simulink model "MX_Gateway"  (Simulink 9.0)
settings: Solver=FixedStepDiscrete  StartTime=0.0  StopTime=100  FixedStep=0.01  RelTol=1e-3
values from neighbouring files (symbols this model uses):
  ACTIVE = Bobcat.Parameter; CreateDefine( ACTIVE                         ,1                          ,'Define' ,'defines.h' )    [defines.m]
  ACTIVE_MODE_ONE = Bobcat.Parameter; CreateDefine( ACTIVE_MODE_ONE                ,19                         ,'Define' ,'defines.h' )    [defines.m]
  AUTHENTICATED = Bobcat.Parameter; CreateDefine( AUTHENTICATED                  ,1                          ,'Define' ,'defines.h' )    [defines.m]
  AUX_EMEA_CONTROLS = Bobcat.Parameter; CreateDefine( AUX_EMEA_CONTROLS  ,1   ,'Define' ,'defines.h' )    [enums.m]
  AUX_FLOW_HIGH = Bobcat.Parameter; CreateDefine( AUX_FLOW_HIGH           ,4  ,'Define' ,'defines.h' )    [enums.m]
  AUX_FLOW_INACTIVE = Bobcat.Parameter; CreateDefine( AUX_FLOW_INACTIVE       ,0  ,'Define' ,'defines.h' )    [enums.m]
  AUX_FLOW_LOW = Bobcat.Parameter; CreateDefine( AUX_FLOW_LOW            ,1  ,'Define' ,'defines.h' )    [enums.m]
  AUX_FLOW_MEDIUM = Bobcat.Parameter; CreateDefine( AUX_FLOW_MEDIUM         ,2  ,'Define' ,'defines.h' )    [enums.m]
  AUX_FLOW_STANDARD = Bobcat.Parameter; CreateDefine( AUX_FLOW_STANDARD       ,3  ,'Define' ,'defines.h' )    [enums.m]
  AUX_NON_FUNCTIONAL = Bobcat.Parameter; CreateDefine( AUX_NON_FUNCTIONAL ,255 ,'Define' ,'defines.h' )    [enums.m]
  BASE_ACTIVE = Bobcat.Parameter; CreateDefine( BASE_ACTIVE                    ,253                        ,'Define' ,'defines.h' )    [defines.m]
  BASE_DETENT = Bobcat.Parameter; CreateDefine( BASE_DETENT                    ,1                          ,'Define' ,'defines.h' )    [defines.m]
  BEEP_NONE = Bobcat.Parameter; CreateDefine( BEEP_NONE                      ,255                        ,'Define' ,'defines.h' )    [defines.m]
  CD_AIR_FILTER_DEBOUNCE_NORMAL = Bobcat.Parameter; CreateAliasCD( CD_AIR_FILTER_DEBOUNCE_NORMAL                        ,uint16(500)                ,'ConstantDataPointer' ,'CONSTANT_DATA' ,'AIR_FILTER_DEBOUNCE_NORMAL')    [cdata.m]    (also in hc12_cdata.m; m4_cdata.m)
  CD_AIR_FILTER_DEBOUNCE_NOT_CONNECTED = Bobcat.Parameter; CreateAliasCD( CD_AIR_FILTER_DEBOUNCE_NOT_CONNECTED                 ,uint16(3000)               ,'ConstantDataPointer' ,'CONSTANT_DATA' ,'AIR_FILTER_DEBOUNCE_NOT_CONNECTED')    [cdata.m]    (also in hc12_cdata.m; m4_cdata.m)
  CD_AIR_FILTER_DEBOUNCE_PLUGGED = Bobcat.Parameter; CreateAliasCD( CD_AIR_FILTER_DEBOUNCE_PLUGGED                       ,uint16(3000)               ,'ConstantDataPointer' ,'CONSTANT_DATA' ,'AIR_FILTER_DEBOUNCE_PLUGGED')    [cdata.m]    (also in hc12_cdata.m; m4_cdata.m)
  CD_AIR_FILTER_FUNCTION = Bobcat.Parameter; CreateAliasCD( CD_AIR_FILTER_FUNCTION                               ,uint8(255)                 ,'ConstantDataPointer' ,'CONSTANT_DATA' ,'AIR_FILTER_FUNCTION')    [cdata.m]    (also in hc12_cdata.m; m4_cdata.m)
  CD_AIR_FILTER_PLUGGED_WEIGHT = Bobcat.Parameter; CreateAliasCD( CD_AIR_FILTER_PLUGGED_WEIGHT                         ,uint8(2)                   ,'ConstantDataPointer' ,'CONSTANT_DATA' ,'AIR_FILTER_PLUGGED_WEIGHT')    [cdata.m]    (also in hc12_cdata.m; m4_cdata.m)
  CD_AIR_FILTER_UNPLUGGED_WEIGHT = Bobcat.Parameter; CreateAliasCD( CD_AIR_FILTER_UNPLUGGED_WEIGHT                       ,uint8(1)                   ,'ConstantDataPointer' ,'CONSTANT_DATA' ,'AIR_FILTER_UNPLUGGED_WEIGHT')    [cdata.m]    (also in hc12_cdata.m; m4_cdata.m)
  CD_AUTOIDLE_ARMBAR_DELAY = Bobcat.Parameter; CreateAliasCD( CD_AUTOIDLE_ARMBAR_DELAY                             ,uint8(2)                   ,'ConstantDataPointer' ,'CONSTANT_DATA' ,'AUTOIDLE_ARMBAR_DELAY')    [cdata.m]    (also in hc12_cdata.m; m4_cdata.m)
  CD_AUTOIDLE_FUNCTION = Bobcat.Parameter; CreateAliasCD( CD_AUTOIDLE_FUNCTION                                 ,uint8(255)                 ,'ConstantDataPointer' ,'CONSTANT_DATA' ,'AUTOIDLE_FUNCTION')    [cdata.m]    (also in hc12_cdata.m; m4_cdata.m)
  CD_AUTOIDLE_IDLE_DELAY = Bobcat.Parameter; CreateAliasCD( CD_AUTOIDLE_IDLE_DELAY                               ,uint8(40)                  ,'ConstantDataPointer' ,'CONSTANT_DATA' ,'AUTOIDLE_IDLE_DELAY')    [cdata.m]    (also in hc12_cdata.m; m4_cdata.m)
  CD_AUTOIDLE_LOW_IDLE_START = Bobcat.Parameter; CreateAliasCD( CD_AUTOIDLE_LOW_IDLE_START                           ,uint8(255)                 ,'ConstantDataPointer' ,'CONSTANT_DATA' ,'AUTOIDLE_LOW_IDLE_START')    [cdata.m]    (also in hc12_cdata.m; m4_cdata.m)
  CD_AUTOIDLE_PRESSURE_OFF = Bobcat.Parameter; CreateAliasCD( CD_AUTOIDLE_PRESSURE_OFF                             ,uint16(300)                ,'ConstantDataPointer' ,'CONSTANT_DATA' ,'AUTOIDLE_PRESSURE_OFF')    [cdata.m]    (also in hc12_cdata.m; m4_cdata.m)
  CD_AUTOIDLE_PRESSURE_ON = Bobcat.Parameter; CreateAliasCD( CD_AUTOIDLE_PRESSURE_ON                              ,uint16(270)                ,'ConstantDataPointer' ,'CONSTANT_DATA' ,'AUTOIDLE_PRESSURE_ON')    [cdata.m]    (also in hc12_cdata.m; m4_cdata.m)
  CD_AUTOSHIFT_FUNCTION = Bobcat.Parameter; CreateAliasCD( CD_AUTOSHIFT_FUNCTION                                ,uint8(255)                 ,'ConstantDataPointer' ,'CONSTANT_DATA' ,'AUTOSHIFT_FUNCTION')    [cdata.m]    (also in hc12_cdata.m; m4_cdata.m)
  CD_AUTOSHIFT_PHIGH1 = Bobcat.Parameter; CreateAliasCD( CD_AUTOSHIFT_PHIGH1                                  ,uint16(205)                ,'ConstantDataPointer' ,'CONSTANT_DATA' ,'AUTOSHIFT_PHIGH1')    [cdata.m]    (also in hc12_cdata.m; m4_cdata.m)
  CD_AUTOSHIFT_PHIGH2 = Bobcat.Parameter; CreateAliasCD( CD_AUTOSHIFT_PHIGH2                                  ,uint16(193)                ,'ConstantDataPointer' ,'CONSTANT_DATA' ,'AUTOSHIFT_PHIGH2')    [cdata.m]    (also in hc12_cdata.m; m4_cdata.m)
  CD_AUTOSHIFT_PHIGH_TEMP1 = Bobcat.Parameter; CreateAliasCD( CD_AUTOSHIFT_PHIGH_TEMP1                             ,int16(70)                  ,'ConstantDataPointer' ,'CONSTANT_DATA' ,'AUTOSHIFT_PHIGH_TEMP1')    [cdata.m]    (also in hc12_cdata.m; m4_cdata.m)
  CD_AUTOSHIFT_PHIGH_TEMP2 = Bobcat.Parameter; CreateAliasCD( CD_AUTOSHIFT_PHIGH_TEMP2                             ,int16(160)                 ,'ConstantDataPointer' ,'CONSTANT_DATA' ,'AUTOSHIFT_PHIGH_TEMP2')    [cdata.m]    (also in hc12_cdata.m; m4_cdata.m)
  CD_AUTOSHIFT_PLOW1 = Bobcat.Parameter; CreateAliasCD( CD_AUTOSHIFT_PLOW1                                   ,uint16(130)                ,'ConstantDataPointer' ,'CONSTANT_DATA' ,'AUTOSHIFT_PLOW1')    [cdata.m]    (also in hc12_cdata.m; m4_cdata.m)
  CD_AUTOSHIFT_PLOW2 = Bobcat.Parameter; CreateAliasCD( CD_AUTOSHIFT_PLOW2                                   ,uint16(100)                ,'ConstantDataPointer' ,'CONSTANT_DATA' ,'AUTOSHIFT_PLOW2')    [cdata.m]    (also in hc12_cdata.m; m4_cdata.m)
  CD_AUTOSHIFT_PLOW_TEMP1 = Bobcat.Parameter; CreateAliasCD( CD_AUTOSHIFT_PLOW_TEMP1                              ,int16(20)                  ,'ConstantDataPointer' ,'CONSTANT_DATA' ,'AUTOSHIFT_PLOW_TEMP1')    [cdata.m]    (also in hc12_cdata.m; m4_cdata.m)
  CD_AUTOSHIFT_PLOW_TEMP2 = Bobcat.Parameter; CreateAliasCD( CD_AUTOSHIFT_PLOW_TEMP2                              ,int16(80)                  ,'ConstantDataPointer' ,'CONSTANT_DATA' ,'AUTOSHIFT_PLOW_TEMP2')    [cdata.m]    (also in hc12_cdata.m; m4_cdata.m)
  CD_AUTOSHIFT_TRAVEL_DELAY = Bobcat.Parameter; CreateAliasCD( CD_AUTOSHIFT_TRAVEL_DELAY                            ,uint8(32)                  ,'ConstantDataPointer' ,'CONSTANT_DATA' ,'AUTOSHIFT_TRAVEL_DELAY')    [cdata.m]    (also in hc12_cdata.m; m4_cdata.m)
  CD_AUX2_CRACKPOINT_CURRENT = Bobcat.Parameter; CreateAliasCD( CD_AUX2_CRACKPOINT_CURRENT                           ,fi(0,0,16,12,'hex','0c28') ,'ConstantDataPointer' ,'CONSTANT_DATA' ,'AUX2_CRACKPOINT_CURRENT')    [cdata.m]    (also in hc12_cdata.m; m4_cdata.m)
  CD_AUX2_FLOW_LOW_FUNCTION = Bobcat.Parameter; CreateAliasCD( CD_AUX2_FLOW_LOW_FUNCTION                            ,uint8(255)                 ,'ConstantDataPointer' ,'CONSTANT_DATA' ,'AUX2_FLOW_LOW_FUNCTION')    [cdata.m]    (also in hc12_cdata.m; m4_cdata.m)
  CD_AUX2_FLOW_MED_FUNCTION = Bobcat.Parameter; CreateAliasCD( CD_AUX2_FLOW_MED_FUNCTION                            ,uint8(255)                 ,'ConstantDataPointer' ,'CONSTANT_DATA' ,'AUX2_FLOW_MED_FUNCTION')    [cdata.m]    (also in hc12_cdata.m; m4_cdata.m)
  CD_AUX2_HI_FLOW_PERCENT = Bobcat.Parameter; CreateAliasCD( CD_AUX2_HI_FLOW_PERCENT                              ,fi(0,0,16,16,'hex','ffff') ,'ConstantDataPointer' ,'CONSTANT_DATA' ,'AUX2_HI_FLOW_PERCENT')    [cdata.m]    (also in hc12_cdata.m; m4_cdata.m)
  CD_AUX2_LOW_FLOW_PERCENT = Bobcat.Parameter; CreateAliasCD( CD_AUX2_LOW_FLOW_PERCENT                             ,fi(0,0,16,16,'hex','7333') ,'ConstantDataPointer' ,'CONSTANT_DATA' ,'AUX2_LOW_FLOW_PERCENT')    [cdata.m]    (also in hc12_cdata.m; m4_cdata.m)
  CD_AUX2_MAX_FLOW_CURRENT = Bobcat.Parameter; CreateAliasCD( CD_AUX2_MAX_FLOW_CURRENT                             ,fi(0,0,16,12,'hex','14cc') ,'ConstantDataPointer' ,'CONSTANT_DATA' ,'AUX2_MAX_FLOW_CURRENT')    [cdata.m]    (also in hc12_cdata.m; m4_cdata.m)
  CD_AUX2_MED_FLOW_PERCENT = Bobcat.Parameter; CreateAliasCD( CD_AUX2_MED_FLOW_PERCENT                             ,fi(0,0,16,16,'hex','9999') ,'ConstantDataPointer' ,'CONSTANT_DATA' ,'AUX2_MED_FLOW_PERCENT')    [cdata.m]    (also in hc12_cdata.m; m4_cdata.m)
  CD_AUX_CRACKPOINT_CURRENT = Bobcat.Parameter; CreateAliasCD( CD_AUX_CRACKPOINT_CURRENT                            ,fi(0,0,16,12,'hex','0000') ,'ConstantDataPointer' ,'CONSTANT_DATA' ,'AUX_CRACKPOINT_CURRENT')    [cdata.m]    (also in hc12_cdata.m; m4_cdata.m)
  CD_AUX_DETENT_FLASH_TIME = Bobcat.Parameter; CreateAliasCD( CD_AUX_DETENT_FLASH_TIME                             ,uint16(72)                 ,'ConstantDataPointer' ,'CONSTANT_DATA' ,'AUX_DETENT_FLASH_TIME')    [cdata.m]    (also in hc12_cdata.m; m4_cdata.m)
  CD_AUX_DETENT_HOLD_TIME = Bobcat.Parameter; CreateAliasCD( CD_AUX_DETENT_HOLD_TIME                              ,uint16(100)                ,'ConstantDataPointer' ,'CONSTANT_DATA' ,'AUX_DETENT_HOLD_TIME')    [cdata.m]    (also in hc12_cdata.m; m4_cdata.m)
  CD_AUX_FULL_ON_AUX1 = Bobcat.Parameter; CreateAliasCD( CD_AUX_FULL_ON_AUX1                                  ,fi(0,0,16,12,'hex','10cd') ,'ConstantDataPointer' ,'CONSTANT_DATA' ,'AUX_FULL_ON_AUX1')    [cdata.m]    (also in hc12_cdata.m; m4_cdata.m)
  CD_AUX_FULL_ON_AUX2 = Bobcat.Parameter; CreateAliasCD( CD_AUX_FULL_ON_AUX2                                  ,fi(0,0,16,12,'hex','139d') ,'ConstantDataPointer' ,'CONSTANT_DATA' ,'AUX_FULL_ON_AUX2')    [cdata.m]    (also in hc12_cdata.m; m4_cdata.m)
  CD_AUX_FULL_ON_AUX3 = Bobcat.Parameter; CreateAliasCD( CD_AUX_FULL_ON_AUX3                                  ,fi(0,0,16,12,'hex','17d7') ,'ConstantDataPointer' ,'CONSTANT_DATA' ,'AUX_FULL_ON_AUX3')    [cdata.m]    (also in hc12_cdata.m; m4_cdata.m)
  CD_AUX_FUNCTION = Bobcat.Parameter; CreateAliasCD( CD_AUX_FUNCTION                                      ,uint8(0)                   ,'ConstantDataPointer' ,'CONSTANT_DATA' ,'AUX_FUNCTION')    [cdata.m]    (also in hc12_cdata.m; m4_cdata.m)
  CD_AUX_RATE_LIMIT_CRACKPOINT = Bobcat.Parameter; CreateAliasCD( CD_AUX_RATE_LIMIT_CRACKPOINT                         ,fi(0,0,16,12,'hex','0800') ,'ConstantDataPointer' ,'CONSTANT_DATA' ,'AUX_RATE_LIMIT_CRACKPOINT')    [cdata.m]    (also in hc12_cdata.m; m4_cdata.m)
  CD_AUX_RATE_LIMIT_CRACKPOINT_RATE = Bobcat.Parameter; CreateAliasCD( CD_AUX_RATE_LIMIT_CRACKPOINT_RATE                    ,fi(0,1,32,12,'int',102400) ,'ConstantDataPointer' ,'CONSTANT_DATA' ,'AUX_RATE_LIMIT_CRACKPOINT_RATE')    [cdata.m]    (also in hc12_cdata.m; m4_cdata.m)
  CD_AUX_RATE_LIMIT_FUNCTION = Bobcat.Parameter; CreateAliasCD( CD_AUX_RATE_LIMIT_FUNCTION                           ,uint8(255)                 ,'ConstantDataPointer' ,'CONSTANT_DATA' ,'AUX_RATE_LIMIT_FUNCTION')    [cdata.m]    (also in hc12_cdata.m; m4_cdata.m)
  CD_AUX_RATE_LIMIT_MAX_ENG_SPD = Bobcat.Parameter; CreateAliasCD( CD_AUX_RATE_LIMIT_MAX_ENG_SPD                        ,uint16(1600)               ,'ConstantDataPointer' ,'CONSTANT_DATA' ,'AUX_RATE_LIMIT_MAX_ENG_SPD')    [cdata.m]    (also in hc12_cdata.m; m4_cdata.m)
  CD_AUX_RATE_LIMIT_MIN_ENG_SPD = Bobcat.Parameter; CreateAliasCD( CD_AUX_RATE_LIMIT_MIN_ENG_SPD                        ,uint16(100)                ,'ConstantDataPointer' ,'CONSTANT_DATA' ,'AUX_RATE_LIMIT_MIN_ENG_SPD')    [cdata.m]    (also in hc12_cdata.m; m4_cdata.m)
  CD_AUX_RATE_LIMIT_NORMAL_RATE = Bobcat.Parameter; CreateAliasCD( CD_AUX_RATE_LIMIT_NORMAL_RATE                        ,fi(0,1,32,12,'int',2048)   ,'ConstantDataPointer' ,'CONSTANT_DATA' ,'AUX_RATE_LIMIT_NORMAL_RATE')    [cdata.m]    (also in hc12_cdata.m; m4_cdata.m)
  CD_AUXPOT_CAL_DEADBAND_HIGH = Bobcat.Parameter; CreateAliasCD( CD_AUXPOT_CAL_DEADBAND_HIGH                          ,uint16(574)                ,'ConstantDataPointer' ,'CONSTANT_DATA' ,'AUXPOT_CAL_DEADBAND_HIGH')    [cdata.m]    (also in hc12_cdata.m; m4_cdata.m)
  CD_AUXPOT_CAL_DEADBAND_LOW = Bobcat.Parameter; CreateAliasCD( CD_AUXPOT_CAL_DEADBAND_LOW                           ,uint16(424)                ,'ConstantDataPointer' ,'CONSTANT_DATA' ,'AUXPOT_CAL_DEADBAND_LOW')    [cdata.m]    (also in hc12_cdata.m; m4_cdata.m)
  CD_AUXPOT_CENTER_RATIO = Bobcat.Parameter; CreateAliasCD( CD_AUXPOT_CENTER_RATIO                               ,uint16(500)                ,'ConstantDataPointer' ,'CONSTANT_DATA' ,'AUXPOT_CENTER_RATIO')    [cdata.m]    (also in hc12_cdata.m; m4_cdata.m)
  CD_AUXPOT_DEADBAND = Bobcat.Parameter; CreateAliasCD( CD_AUXPOT_DEADBAND                                   ,uint8(38)                  ,'ConstantDataPointer' ,'CONSTANT_DATA' ,'AUXPOT_DEADBAND')    [cdata.m]    (also in hc12_cdata.m; m4_cdata.m)
  CD_AUXPOT_FUNCTION = Bobcat.Parameter; CreateAliasCD( CD_AUXPOT_FUNCTION                                   ,uint8(0)                   ,'ConstantDataPointer' ,'CONSTANT_DATA' ,'AUXPOT_FUNCTION')    [cdata.m]    (also in hc12_cdata.m; m4_cdata.m)
  CD_AUXPOT_RATIO_GAIN = Bobcat.Parameter; CreateAliasCD( CD_AUXPOT_RATIO_GAIN                                 ,uint16(1000)               ,'ConstantDataPointer' ,'CONSTANT_DATA' ,'AUXPOT_RATIO_GAIN')    [cdata.m]    (also in hc12_cdata.m; m4_cdata.m)
  CD_CAN_THROTTLE_FUNCTION = Bobcat.Parameter; CreateAliasCD( CD_CAN_THROTTLE_FUNCTION                             ,uint8(255)                 ,'ConstantDataPointer' ,'CONSTANT_DATA' ,'CAN_THROTTLE_FUNCTION')    [cdata.m]    (also in hc12_cdata.m; m4_cdata.m)
  CD_CAN_THROTTLE_REVERSE = Bobcat.Parameter; CreateAliasCD( CD_CAN_THROTTLE_REVERSE                              ,uint8(255)                 ,'ConstantDataPointer' ,'CONSTANT_DATA' ,'CAN_THROTTLE_REVERSE')    [cdata.m]    (also in hc12_cdata.m; m4_cdata.m)
  CD_CONSOLE_DEBOUNCE_ABSENT = Bobcat.Parameter; CreateAliasCD( CD_CONSOLE_DEBOUNCE_ABSENT                           ,uint16(12)                 ,'ConstantDataPointer' ,'CONSTANT_DATA' ,'CONSOLE_DEBOUNCE_ABSENT')    [cdata.m]    (also in hc12_cdata.m; m4_cdata.m)
  CD_CONSOLE_DEBOUNCE_OORH = Bobcat.Parameter; CreateAliasCD( CD_CONSOLE_DEBOUNCE_OORH                             ,uint16(12)                 ,'ConstantDataPointer' ,'CONSTANT_DATA' ,'CONSOLE_DEBOUNCE_OORH')    [cdata.m]    (also in hc12_cdata.m; m4_cdata.m)
  CD_CONSOLE_DEBOUNCE_OORL = Bobcat.Parameter; CreateAliasCD( CD_CONSOLE_DEBOUNCE_OORL                             ,uint16(12)                 ,'ConstantDataPointer' ,'CONSTANT_DATA' ,'CONSOLE_DEBOUNCE_OORL')    [cdata.m]    (also in hc12_cdata.m; m4_cdata.m)
  CD_CONSOLE_DEBOUNCE_PRESENT = Bobcat.Parameter; CreateAliasCD( CD_CONSOLE_DEBOUNCE_PRESENT                          ,uint16(12)                 ,'ConstantDataPointer' ,'CONSTANT_DATA' ,'CONSOLE_DEBOUNCE_PRESENT')    [cdata.m]    (also in hc12_cdata.m; m4_cdata.m)
  CD_CONSOLE_FUNCTION = Bobcat.Parameter; CreateAliasCD( CD_CONSOLE_FUNCTION                                  ,uint8(0)                   ,'ConstantDataPointer' ,'CONSTANT_DATA' ,'CONSOLE_FUNCTION')    [cdata.m]    (also in hc12_cdata.m; m4_cdata.m)
  CD_CONSOLE_OORH = Bobcat.Parameter; CreateAliasCD( CD_CONSOLE_OORH                                      ,uint16(740)                ,'ConstantDataPointer' ,'CONSTANT_DATA' ,'CONSOLE_OORH')    [cdata.m]    (also in hc12_cdata.m; m4_cdata.m)
  CD_CONSOLE_OORL = Bobcat.Parameter; CreateAliasCD( CD_CONSOLE_OORL                                      ,uint16(10)                 ,'ConstantDataPointer' ,'CONSTANT_DATA' ,'CONSOLE_OORL')    [cdata.m]    (also in hc12_cdata.m; m4_cdata.m)
  CD_CONSOLE_PRESENT_HIGH = Bobcat.Parameter; CreateAliasCD( CD_CONSOLE_PRESENT_HIGH                              ,uint16(515)                ,'ConstantDataPointer' ,'CONSTANT_DATA' ,'CONSOLE_PRESENT_HIGH')    [cdata.m]    (also in hc12_cdata.m; m4_cdata.m)
  CD_CONSOLE_PRESENT_LOW = Bobcat.Parameter; CreateAliasCD( CD_CONSOLE_PRESENT_LOW                               ,uint16(375)                ,'ConstantDataPointer' ,'CONSTANT_DATA' ,'CONSOLE_PRESENT_LOW')    [cdata.m]    (also in hc12_cdata.m; m4_cdata.m)
  CD_DELUXE_GEN5_5_FUNCTION = Bobcat.Parameter; CreateAliasCD( CD_DELUXE_GEN5_5_FUNCTION                            ,uint8(255)                 ,'ConstantDataPointer' ,'CONSTANT_DATA' ,'DELUXE_GEN5_5_FUNCTION')    [cdata.m]    (also in hc12_cdata.m; m4_cdata.m)
  CD_DELUXE_GEN5_FUNCTION = Bobcat.Parameter; CreateAliasCD( CD_DELUXE_GEN5_FUNCTION                              ,uint8(255)                 ,'ConstantDataPointer' ,'CONSTANT_DATA' ,'DELUXE_GEN5_FUNCTION')    [cdata.m]    (also in hc12_cdata.m; m4_cdata.m)
  CD_DERATE_ENGINE_SPEED_DEBOUNCE_HIGH = Bobcat.Parameter; CreateAliasCD( CD_DERATE_ENGINE_SPEED_DEBOUNCE_HIGH                 ,uint16(200)                ,'ConstantDataPointer' ,'CONSTANT_DATA' ,'DERATE_ENGINE_SPEED_DEBOUNCE_HIGH')    [cdata.m]    (also in hc12_cdata.m; m4_cdata.m)
  CD_DERATE_ENGINE_SPEED_DEBOUNCE_SHUTDOWN = Bobcat.Parameter; CreateAliasCD( CD_DERATE_ENGINE_SPEED_DEBOUNCE_SHUTDOWN             ,uint16(1000)               ,'ConstantDataPointer' ,'CONSTANT_DATA' ,'DERATE_ENGINE_SPEED_DEBOUNCE_SHUTDOWN')    [cdata.m]    (also in hc12_cdata.m; m4_cdata.m)
  CD_DERATE_ENGINE_SPEED_HIGH_OFFSET = Bobcat.Parameter; CreateAliasCD( CD_DERATE_ENGINE_SPEED_HIGH_OFFSET                   ,uint16(200)                ,'ConstantDataPointer' ,'CONSTANT_DATA' ,'DERATE_ENGINE_SPEED_HIGH_OFFSET')    [cdata.m]    (also in hc12_cdata.m; m4_cdata.m)
  CD_DERATE_METHOD = Bobcat.Parameter; CreateAliasCD( CD_DERATE_METHOD                                     ,uint8(0)                   ,'ConstantDataPointer' ,'CONSTANT_DATA' ,'DERATE_METHOD')    [cdata.m]    (also in hc12_cdata.m; m4_cdata.m)
  CD_DUMP_VALVE_FUNCTION = Bobcat.Parameter; CreateAliasCD( CD_DUMP_VALVE_FUNCTION                               ,uint8(255)                 ,'ConstantDataPointer' ,'CONSTANT_DATA' ,'DUMP_VALVE_FUNCTION')    [cdata.m]    (also in hc12_cdata.m; m4_cdata.m)
  CD_DUMP_VALVE_ON_CURRENT = Bobcat.Parameter; CreateAliasCD( CD_DUMP_VALVE_ON_CURRENT                             ,fi(0,0,16,12,'hex','ffff') ,'ConstantDataPointer' ,'CONSTANT_DATA' ,'DUMP_VALVE_ON_CURRENT')    [cdata.m]    (also in hc12_cdata.m; m4_cdata.m)
  CD_ECO_MODE_THROTTLE_REDUCTION = Bobcat.Parameter; CreateAliasCD( CD_ECO_MODE_THROTTLE_REDUCTION                       ,uint16(850)                ,'ConstantDataPointer' ,'CONSTANT_DATA' ,'ECO_MODE_THROTTLE_REDUCTION')    [cdata.m]    (also in hc12_cdata.m; m4_cdata.m)
  CD_ECT_FILTER_GAIN = Bobcat.Parameter; CreateAliasCD( CD_ECT_FILTER_GAIN                                   ,uint8(6)                   ,'ConstantDataPointer' ,'CONSTANT_DATA' ,'ECT_FILTER_GAIN')    [cdata.m]    (also in hc12_cdata.m; m4_cdata.m)
  CD_ECU_FUNCTION = Bobcat.Parameter; CreateAliasCD( CD_ECU_FUNCTION                                      ,uint8(255)                 ,'ConstantDataPointer' ,'CONSTANT_DATA' ,'ECU_FUNCTION')    [cdata.m]    (also in hc12_cdata.m; m4_cdata.m)
  CD_ECU_HOLD_DEBOUNCE_ERROR_ON = Bobcat.Parameter; CreateAliasCD( CD_ECU_HOLD_DEBOUNCE_ERROR_ON                        ,uint16(20)                 ,'ConstantDataPointer' ,'CONSTANT_DATA' ,'ECU_HOLD_DEBOUNCE_ERROR_ON')    [cdata.m]    (also in hc12_cdata.m; m4_cdata.m)
  CD_EMEA_CONTROLS_HOLD_TIME = Bobcat.Parameter; CreateAliasCD( CD_EMEA_CONTROLS_HOLD_TIME                           ,uint16(30)                 ,'ConstantDataPointer' ,'CONSTANT_DATA' ,'EMEA_CONTROLS_HOLD_TIME')    [cdata.m]    (also in hc12_cdata.m; m4_cdata.m)
  CD_EMEA_CONTROLS_OPTION_A = Bobcat.Parameter; CreateAliasCD( CD_EMEA_CONTROLS_OPTION_A                            ,uint8(255)                 ,'ConstantDataPointer' ,'CONSTANT_DATA' ,'EMEA_CONTROLS_OPTION_A')    [cdata.m]    (also in hc12_cdata.m; m4_cdata.m)
  CD_EMEA_CONTROLS_OPTION_C = Bobcat.Parameter; CreateAliasCD( CD_EMEA_CONTROLS_OPTION_C                            ,uint8(255)                 ,'ConstantDataPointer' ,'CONSTANT_DATA' ,'EMEA_CONTROLS_OPTION_C')    [cdata.m]    (also in hc12_cdata.m; m4_cdata.m)
  CD_EMEA_CONTROLS_REVERSE = Bobcat.Parameter; CreateAliasCD( CD_EMEA_CONTROLS_REVERSE                             ,uint8(255)                 ,'ConstantDataPointer' ,'CONSTANT_DATA' ,'EMEA_CONTROLS_REVERSE')    [cdata.m]    (also in hc12_cdata.m; m4_cdata.m)
  CD_EMEA_ELECTRIC_VERSION = Bobcat.Parameter; CreateAliasCD( CD_EMEA_ELECTRIC_VERSION                             ,uint8(0)                   ,'ConstantDataPointer' ,'CONSTANT_DATA' ,'EMEA_ELECTRIC_VERSION')    [cdata.m]    (also in hc12_cdata.m; m4_cdata.m)
  CD_EMEA_POT_CAL_DEADBAND_HIGH = Bobcat.Parameter; CreateAliasCD( CD_EMEA_POT_CAL_DEADBAND_HIGH                        ,uint16(574)                ,'ConstantDataPointer' ,'CONSTANT_DATA' ,'EMEA_POT_CAL_DEADBAND_HIGH')    [cdata.m]    (also in hc12_cdata.m; m4_cdata.m)
  CD_EMEA_POT_CAL_DEADBAND_LOW = Bobcat.Parameter; CreateAliasCD( CD_EMEA_POT_CAL_DEADBAND_LOW                         ,uint16(424)                ,'ConstantDataPointer' ,'CONSTANT_DATA' ,'EMEA_POT_CAL_DEADBAND_LOW')    [cdata.m]    (also in hc12_cdata.m; m4_cdata.m)
  CD_ENGINE_RPM_FACTOR = Bobcat.Parameter; CreateAliasCD( CD_ENGINE_RPM_FACTOR                                 ,uint8(35)                  ,'ConstantDataPointer' ,'CONSTANT_DATA' ,'ENGINE_RPM_FACTOR')    [cdata.m]    (also in hc12_cdata.m; m4_cdata.m)
  CD_ENGINE_SPEED_FILTER_FAST_VALUE = Bobcat.Parameter; CreateAliasCD( CD_ENGINE_SPEED_FILTER_FAST_VALUE                    ,uint8(1)                   ,'ConstantDataPointer' ,'CONSTANT_DATA' ,'ENGINE_SPEED_FILTER_FAST_VALUE')    [cdata.m]    (also in hc12_cdata.m; m4_cdata.m)
  CD_ENGINE_SPEED_FILTER_SLOW_VALUE = Bobcat.Parameter; CreateAliasCD( CD_ENGINE_SPEED_FILTER_SLOW_VALUE                    ,uint8(4)                   ,'ConstantDataPointer' ,'CONSTANT_DATA' ,'ENGINE_SPEED_FILTER_SLOW_VALUE')    [cdata.m]    (also in hc12_cdata.m; m4_cdata.m)
  CD_ENGINE_SPEED_RUNNING = Bobcat.Parameter; CreateAliasCD( CD_ENGINE_SPEED_RUNNING                              ,uint16(800)                ,'ConstantDataPointer' ,'CONSTANT_DATA' ,'ENGINE_SPEED_RUNNING')    [cdata.m]    (also in hc12_cdata.m; m4_cdata.m)
  CD_ENGINE_START_DELAY_DEBOUNCE_RPM_NO_SIGNAL = Bobcat.Parameter; CreateAliasCD( CD_ENGINE_START_DELAY_DEBOUNCE_RPM_NO_SIGNAL         ,uint16(0)                  ,'ConstantDataPointer' ,'CONSTANT_DATA' ,'ENGINE_START_DELAY_DEBOUNCE_RPM_NO_SIGNAL')    [cdata.m]    (also in hc12_cdata.m; m4_cdata.m)
  CD_ENGINE_START_DELAY_OFF_TIME = Bobcat.Parameter; CreateAliasCD( CD_ENGINE_START_DELAY_OFF_TIME                       ,uint16(0)                  ,'ConstantDataPointer' ,'CONSTANT_DATA' ,'ENGINE_START_DELAY_OFF_TIME')    [cdata.m]    (also in hc12_cdata.m; m4_cdata.m)
  CD_ENGINE_START_DELAY_RPM_NO_SIGNAL = Bobcat.Parameter; CreateAliasCD( CD_ENGINE_START_DELAY_RPM_NO_SIGNAL                  ,uint16(0)                  ,'ConstantDataPointer' ,'CONSTANT_DATA' ,'ENGINE_START_DELAY_RPM_NO_SIGNAL')    [cdata.m]    (also in hc12_cdata.m; m4_cdata.m)
  CD_EOP_DEBOUNCE_EXTREMELY_LOW = Bobcat.Parameter; CreateAliasCD( CD_EOP_DEBOUNCE_EXTREMELY_LOW                        ,uint16(50)                 ,'ConstantDataPointer' ,'CONSTANT_DATA' ,'EOP_DEBOUNCE_EXTREMELY_LOW')    [cdata.m]    (also in hc12_cdata.m; m4_cdata.m)
  CD_EOP_DEBOUNCE_NORMAL = Bobcat.Parameter; CreateAliasCD( CD_EOP_DEBOUNCE_NORMAL                               ,uint16(50)                 ,'ConstantDataPointer' ,'CONSTANT_DATA' ,'EOP_DEBOUNCE_NORMAL')    [cdata.m]    (also in hc12_cdata.m; m4_cdata.m)
  CD_EOP_DEBOUNCE_SHUTDOWN = Bobcat.Parameter; CreateAliasCD( CD_EOP_DEBOUNCE_SHUTDOWN                             ,uint16(100)                ,'ConstantDataPointer' ,'CONSTANT_DATA' ,'EOP_DEBOUNCE_SHUTDOWN')    [cdata.m]    (also in hc12_cdata.m; m4_cdata.m)
  CD_EOP_FILTER_VALUE = Bobcat.Parameter; CreateAliasCD( CD_EOP_FILTER_VALUE                                  ,uint8(3)                   ,'ConstantDataPointer' ,'CONSTANT_DATA' ,'EOP_FILTER_VALUE')    [cdata.m]    (also in hc12_cdata.m; m4_cdata.m)
  CD_EOP_FUNCTION = Bobcat.Parameter; CreateAliasCD( CD_EOP_FUNCTION                                      ,uint8(1)                   ,'ConstantDataPointer' ,'CONSTANT_DATA' ,'EOP_FUNCTION')    [cdata.m]    (also in hc12_cdata.m; m4_cdata.m)
  CD_EOP_SWITCH_GAUGE_VALUE = Bobcat.Parameter; CreateAliasCD( CD_EOP_SWITCH_GAUGE_VALUE                            ,uint16(125)                ,'ConstantDataPointer' ,'CONSTANT_DATA' ,'EOP_SWITCH_GAUGE_VALUE')    [cdata.m]    (also in hc12_cdata.m; m4_cdata.m)
  CD_EOP_SWITCH_LOW = Bobcat.Parameter; CreateAliasCD( CD_EOP_SWITCH_LOW                                    ,uint16(50)                 ,'ConstantDataPointer' ,'CONSTANT_DATA' ,'EOP_SWITCH_LOW')    [cdata.m]    (also in hc12_cdata.m; m4_cdata.m)
  CD_EOP_SWITCH_PRESSURE_VALUE = Bobcat.Parameter; CreateAliasCD( CD_EOP_SWITCH_PRESSURE_VALUE                         ,uint16(40)                 ,'ConstantDataPointer' ,'CONSTANT_DATA' ,'EOP_SWITCH_PRESSURE_VALUE')    [cdata.m]    (also in hc12_cdata.m; m4_cdata.m)
  CD_EOT_DEBOUNCE_EXTREMELY_LOW = Bobcat.Parameter; CreateAliasCD( CD_EOT_DEBOUNCE_EXTREMELY_LOW                        ,uint16(2)                  ,'ConstantDataPointer' ,'CONSTANT_DATA' ,'EOT_DEBOUNCE_EXTREMELY_LOW')    [cdata.m]    (also in hc12_cdata.m; m4_cdata.m)
  CD_EOT_DEBOUNCE_LOW = Bobcat.Parameter; CreateAliasCD( CD_EOT_DEBOUNCE_LOW                                  ,uint16(2)                  ,'ConstantDataPointer' ,'CONSTANT_DATA' ,'EOT_DEBOUNCE_LOW')    [cdata.m]    (also in hc12_cdata.m; m4_cdata.m)
  CD_EOT_DEBOUNCE_NORMAL = Bobcat.Parameter; CreateAliasCD( CD_EOT_DEBOUNCE_NORMAL                               ,uint16(2)                  ,'ConstantDataPointer' ,'CONSTANT_DATA' ,'EOT_DEBOUNCE_NORMAL')    [cdata.m]    (also in hc12_cdata.m; m4_cdata.m)
  CD_EOT_EXTREMELY_LOW = Bobcat.Parameter; CreateAliasCD( CD_EOT_EXTREMELY_LOW                                 ,uint16(81)                 ,'ConstantDataPointer' ,'CONSTANT_DATA' ,'EOT_EXTREMELY_LOW')    [cdata.m]    (also in hc12_cdata.m; m4_cdata.m)
  CD_EOT_FUNCTION = Bobcat.Parameter; CreateAliasCD( CD_EOT_FUNCTION                                      ,uint8(255)                 ,'ConstantDataPointer' ,'CONSTANT_DATA' ,'EOT_FUNCTION')    [cdata.m]    (also in hc12_cdata.m; m4_cdata.m)
  CD_EOT_LOW = Bobcat.Parameter; CreateAliasCD( CD_EOT_LOW                                           ,uint16(90)                 ,'ConstantDataPointer' ,'CONSTANT_DATA' ,'EOT_LOW')    [cdata.m]    (also in hc12_cdata.m; m4_cdata.m)
  CD_EOT_TEMPERATURE_HYSTERESIS = Bobcat.Parameter; CreateAliasCD( CD_EOT_TEMPERATURE_HYSTERESIS                        ,uint16(2)                  ,'ConstantDataPointer' ,'CONSTANT_DATA' ,'EOT_TEMPERATURE_HYSTERESIS')    [cdata.m]    (also in hc12_cdata.m; m4_cdata.m)
  CD_FAST_TRAVEL_FUNCTION = Bobcat.Parameter; CreateAliasCD( CD_FAST_TRAVEL_FUNCTION                              ,uint8(255)                 ,'ConstantDataPointer' ,'CONSTANT_DATA' ,'FAST_TRAVEL_FUNCTION')    [cdata.m]    (also in hc12_cdata.m; m4_cdata.m)
  CD_FAST_TRAVEL_THROTTLE_DELAY = Bobcat.Parameter; CreateAliasCD( CD_FAST_TRAVEL_THROTTLE_DELAY                        ,uint16(10)                 ,'ConstantDataPointer' ,'CONSTANT_DATA' ,'FAST_TRAVEL_THROTTLE_DELAY')    [cdata.m]    (also in hc12_cdata.m; m4_cdata.m)
  CD_FAST_TRAVEL_THROTTLE_LIMIT = Bobcat.Parameter; CreateAliasCD( CD_FAST_TRAVEL_THROTTLE_LIMIT                        ,uint16(1000)               ,'ConstantDataPointer' ,'CONSTANT_DATA' ,'FAST_TRAVEL_THROTTLE_LIMIT')    [cdata.m]    (also in hc12_cdata.m; m4_cdata.m)
  CD_FLYWHEEL_TEETH = Bobcat.Parameter; CreateAliasCD( CD_FLYWHEEL_TEETH                                    ,uint8(83)                  ,'ConstantDataPointer' ,'CONSTANT_DATA' ,'FLYWHEEL_TEETH')    [cdata.m]    (also in hc12_cdata.m; m4_cdata.m)
  CD_FUEL_LEVEL_FILTER_FAST_VALUE = Bobcat.Parameter; CreateAliasCD( CD_FUEL_LEVEL_FILTER_FAST_VALUE                      ,uint8(5)                   ,'ConstantDataPointer' ,'CONSTANT_DATA' ,'FUEL_LEVEL_FILTER_FAST_VALUE')    [cdata.m]    (also in hc12_cdata.m; m4_cdata.m)
  CD_FUEL_LEVEL_FILTER_SLOW_VALUE = Bobcat.Parameter; CreateAliasCD( CD_FUEL_LEVEL_FILTER_SLOW_VALUE                      ,uint8(10)                  ,'ConstantDataPointer' ,'CONSTANT_DATA' ,'FUEL_LEVEL_FILTER_SLOW_VALUE')    [cdata.m]    (also in hc12_cdata.m; m4_cdata.m)
  CD_FUEL_PRIMARY_FEEDBACK_FUNCTION = Bobcat.Parameter; CreateAliasCD( CD_FUEL_PRIMARY_FEEDBACK_FUNCTION                    ,uint8(0)                   ,'ConstantDataPointer' ,'CONSTANT_DATA' ,'FUEL_PRIMARY_FEEDBACK_FUNCTION')    [cdata.m]    (also in hc12_cdata.m; m4_cdata.m)
  ... (+246 more)
undefined symbols (not defined in the file or in its folder): sigbuilder_block
undefined symbols (not defined in the file): CD_FUEL_RESOLUTION_TIMER
libraries: simulink x186, MX_Library x107
(part 30 of 48)

== stateflow chart MX_Gtwy_Control/System/Controller_State/Controller_State ==
  state SECONDARY [parallel]
    settings: executionOrder=1
    state NON_FUNCTIONAL
      en:
      state_CommSecondary = INACTIVE;
    state NO_COMMUNICATION
      en:
      state_CommSecondary = NO_COMMUNICATION;
    state NORMAL
      en:
      state_CommSecondary = NORMAL;
    (default) -> SECONDARY.NON_FUNCTIONAL
    SECONDARY.NON_FUNCTIONAL -> SECONDARY.NO_COMMUNICATION [order 2] : [(CD_SECONDARY_FUNCTION != NON_FUNCTIONAL)...
        | && after(CD_SECONDARY_DEBOUNCE_MISSING, msec)...
        | && (state_System == SYS_NORMAL)]
    SECONDARY.NON_FUNCTIONAL -> SECONDARY.NORMAL [order 1] : [(CD_SECONDARY_FUNCTION != NON_FUNCTIONAL)...
        | && (msgAge_Secondary < CD_SECONDARY_DEBOUNCE_MISSING)]
    SECONDARY.NORMAL -> SECONDARY.NO_COMMUNICATION : [(state_Engine != ENGINE_CRANKING)...
        | && (state_System == SYS_NORMAL)...
        | && (msgAge_Secondary >= CD_SECONDARY_DEBOUNCE_MISSING)...
        | && (state_SwitchedPwrOutput == NORMAL)...
        | && (state_SwitchedPwrRelay == NORMAL)]
  state THROTTLE [parallel]
    settings: executionOrder=2
    state NON_FUNCTIONAL
      en:
      state_CommThrottle = INACTIVE;
    state NO_COMMUNICATION
      en:
      state_CommThrottle = NO_COMMUNICATION;
    state NORMAL
      en:
      state_CommThrottle = NORMAL;
    (default) -> THROTTLE.NON_FUNCTIONAL
    THROTTLE.NON_FUNCTIONAL -> THROTTLE.NORMAL : [(CD_THROTTLE_TYPE == ACTUATOR)...
        | || (CD_THROTTLE_TYPE == CAN_ACTUATOR)]
    THROTTLE.NORMAL -> THROTTLE.NO_COMMUNICATION : [(state_Engine != ENGINE_CRANKING)...
        | && (state_System == SYS_NORMAL)...
        | && (msgAge_Throttle >= CD_THROTTLE_DEBOUNCE_MISSING)...
        | && (state_SwitchedPwrOutput == NORMAL)...
        | && (state_SwitchedPwrRelay == NORMAL)]
  state ENGINE [parallel]
    settings: executionOrder=3
    state NON_FUNCTIONAL
      en:
      state_CommECU = INACTIVE;
    state NORMAL
      en:
      state_CommECU = NORMAL;
    state NO_COMMUNICATION
      en:
      state_CommECU = NO_COMMUNICATION;
    note: msgAge_ECU gets set to 0 when engine state == ENGINE CRANKING in ap_can.c file
    (default) -> ENGINE.NON_FUNCTIONAL
    ENGINE.NON_FUNCTIONAL -> ENGINE.NO_COMMUNICATION [order 2] : [(CD_ECU_FUNCTION != NON_FUNCTIONAL)...
        | && (((msgAge_ECU >= CD_ECU_DEBOUNCE_MISSING_ENGINE_NOT_RUNNING) && (state_Engine == ENGINE_NOT_RUNNING))...
        |         || ((msgAge_ECU >= CD_ECU_DEBOUNCE_MISSING_ENGINE_RUNNING) && (state_Engine == ENGINE_RUNNING)))...
        | && (state_System == SYS_NORMAL)...
        | && (msgAge_ECU != 65535)...
        | && (error_FuelSec == NORMAL)...
        | && (state_ECUHold == ACTIVE)]
    ENGINE.NON_FUNCTIONAL -> ENGINE.NORMAL [order 1] : [(CD_ECU_FUNCTION != NON_FUNCTIONAL)...
        | && (msgAge_ECU < CD_ECU_DEBOUNCE_MISSING_ENGINE_RUNNING)]
    ENGINE.NORMAL -> ENGINE.NO_COMMUNICATION : [(state_System == SYS_NORMAL)...
        | && (((msgAge_ECU >= CD_ECU_DEBOUNCE_MISSING_ENGINE_NOT_RUNNING) && (state_Engine == ENGINE_NOT_RUNNING))...
        |         || ((msgAge_ECU >= CD_ECU_DEBOUNCE_MISSING_ENGINE_RUNNING) && (state_Engine == ENGINE_RUNNING)))...
        | && (error_FuelSec == NORMAL)...
        | && (state_ECUHold == ACTIVE)]
  state STD_PANEL [parallel]
    settings: executionOrder=4
    state NON_FUNCTIONAL
      en:
      state_CommStdPanel = INACTIVE;
    state NO_COMMUNICATION
      en:
      state_CommStdPanel = NO_COMMUNICATION;
    state NORMAL
      en:
      state_CommStdPanel = NORMAL;
    (default) -> STD_PANEL.NON_FUNCTIONAL
    STD_PANEL.NON_FUNCTIONAL -> STD_PANEL.NORMAL : [(ee_DeluxeG5 != ACTIVE)...
        | && flag_InitEERead...
        | && (CD_DELUXE_GEN5_5_FUNCTION == NON_FUNCTIONAL)]
    STD_PANEL.NORMAL -> STD_PANEL.NO_COMMUNICATION : [(state_Engine != ENGINE_CRANKING)...
        | && (state_System == SYS_NORMAL)...
        | && (msgAge_StdPanel >= CD_PANEL_DEBOUNCE_MISSING)...
        | && (state_SwitchedPwrOutput == NORMAL)...
        | && (state_SwitchedPwrRelay == NORMAL)]
  state JOG_SHUTTLE [parallel]
    settings: executionOrder=5
    state NON_FUNCTIONAL
      en:
      state_CommJogShuttle = INACTIVE;
    state NO_COMMUNICATION
      en:
      state_CommJogShuttle = NO_COMMUNICATION;
    state NORMAL
      en:
      state_CommJogShuttle = NORMAL;
    (default) -> JOG_SHUTTLE.NON_FUNCTIONAL
    JOG_SHUTTLE.NON_FUNCTIONAL -> JOG_SHUTTLE.NORMAL : [(CD_DELUXE_GEN5_5_FUNCTION != NON_FUNCTIONAL)]
    JOG_SHUTTLE.NORMAL -> JOG_SHUTTLE.NO_COMMUNICATION : [(state_Engine != ENGINE_CRANKING)...
        | && (state_System == SYS_NORMAL)...
        | && (msgAge_JogShuttle >= CD_JOG_SHUTTLE_DEBOUNCE_MISSING)...
        | && (state_SwitchedPwrOutput == NORMAL)...
        | && (state_SwitchedPwrRelay == NORMAL)]
  state DELUXE_G55 [parallel]
    settings: executionOrder=6
    state NON_FUNCTIONAL
      en:
      state_CommDeluxeG55 = INACTIVE;
    state FUNCTIONAL
      state INACTIVE
        en:
        state_CommDeluxeG55 = INACTIVE;
      state EVALUATE
      state NORMAL
        en:
        state_CommDeluxeG55 = NORMAL;
      state NO_COMMUNICATION
        en:
        state_CommDeluxeG55 = NO_COMMUNICATION;
      (default) -> INACTIVE
      INACTIVE -> EVALUATE : [(state_System == SYS_NORMAL)...
          | && (state_SwitchedPwrOutput == NORMAL)...
          | && (state_SwitchedPwrRelay == NORMAL)]
      EVALUATE -> INACTIVE [order 4] : [(state_System != SYS_NORMAL)...
          | || (state_SwitchedPwrOutput != NORMAL)...
          | || (state_SwitchedPwrRelay != NORMAL)]
      EVALUATE -> DELUXE_G55.FUNCTIONAL.NORMAL [order 1] : [(msgAge_DeluxeG55 < CD_DELUXE_G55_COMM_DEBOUNCE_RUNNING)]
      DELUXE_G55.FUNCTIONAL.NORMAL -> EVALUATE : [(msgAge_DeluxeG55 >= CD_DELUXE_G55_COMM_DEBOUNCE_RUNNING)]
      DELUXE_G55.FUNCTIONAL.NO_COMMUNICATION -> DELUXE_G55.FUNCTIONAL.NORMAL : [(msgAge_DeluxeG55 < CD_DELUXE_G55_COMM_DEBOUNCE_RUNNING)]
      EVALUATE -> DELUXE_G55.FUNCTIONAL.NO_COMMUNICATION [order 2] : [(state_Engine == ENGINE_RUNNING)...
          | && (msgAge_DeluxeG55 >= CD_DELUXE_G55_COMM_DEBOUNCE_RUNNING)]
      EVALUATE -> DELUXE_G55.FUNCTIONAL.NO_COMMUNICATION [order 3] : [(state_Engine == ENGINE_NOT_RUNNING)...
          | && (msgAge_DeluxeG55 >= CD_DELUXE_G55_COMM_DEBOUNCE_NOT_RUNNING)]
    (default) -> DELUXE_G55.NON_FUNCTIONAL
    DELUXE_G55.NON_FUNCTIONAL -> FUNCTIONAL : [(CD_DELUXE_GEN5_5_FUNCTION != NON_FUNCTIONAL)]
  state KEYLESS_ENTRY [parallel]
    settings: executionOrder=7
    state NON_FUNCTIONAL
      en:
      state_CommKeylessEntry = INACTIVE;
    state NO_COMMUNICATION
      en:
      state_CommKeylessEntry = NO_COMMUNICATION;
    state NORMAL
      en:
      state_CommKeylessEntry = NORMAL;
    (default) -> KEYLESS_ENTRY.NON_FUNCTIONAL
    KEYLESS_ENTRY.NON_FUNCTIONAL -> KEYLESS_ENTRY.NORMAL : [(CD_KEYLESS_ENTRY_FUNCTION != NON_FUNCTIONAL)]
    KEYLESS_ENTRY.NORMAL -> KEYLESS_ENTRY.NO_COMMUNICATION : [(state_Engine != ENGINE_CRANKING)...
        | && (state_System == SYS_NORMAL)...
        | && (msgAge_KeylessEntry >= CD_KEYLESS_ENTRY_DEBOUNCE_MISSING)...
        | && (state_SwitchedPwrOutput == NORMAL)...
        | && (state_SwitchedPwrRelay == NORMAL)]
  data: state_Engine input uint8; NO_COMMUNICATION parameter uint8; INACTIVE parameter uint8; NORMAL parameter uint8; ENGINE_RUNNING parameter uint8; SYS_NORMAL parameter uint8; state_System input uint8; state_SwitchedPwrOutput input uint8; state_SwitchedPwrRelay input uint8; msgAge_Secondary input uint16; state_CommSecondary output uint8; CD_SECONDARY_DEBOUNCE_MISSING parameter uint16; CD_SECONDARY_FUNCTION parameter uint8; NON_FUNCTIONAL parameter uint8; msgAge_ECU input uint16; state_CommECU output uint8; CD_ECU_FUNCTION parameter uint8; error_FuelSec input uint8; state_ECUHold input uint8; ACTIVE parameter uint8; CD_THROTTLE_DEBOUNCE_MISSING parameter uint16; CD_THROTTLE_TYPE parameter uint8; msgAge_Throttle input uint16; state_CommThrottle output uint8; ACTUATOR parameter uint8; ENGINE_CRANKING parameter uint8; CD_ECU_DEBOUNCE_MISSING_ENGINE_NOT_RUNNING parameter uint16; CD_ECU_DEBOUNCE_MISSING_ENGINE_RUNNING parameter uint16; ENGINE_NOT_RUNNING parameter uint8; CAN_ACTUATOR parameter uint8; CD_DELUXE_GEN5_5_FUNCTION parameter uint8; CD_PANEL_DEBOUNCE_MISSING parameter uint16; CD_DELUXE_G55_COMM_DEBOUNCE_NOT_RUNNING parameter uint16; CD_JOG_SHUTTLE_DEBOUNCE_MISSING parameter uint16; msgAge_StdPanel input uint16; msgAge_JogShuttle input uint16; msgAge_DeluxeG55 input uint16; state_CommStdPanel output uint8; state_CommJogShuttle output uint8; state_CommDeluxeG55 output uint8; ee_DeluxeG5 data_store_memory double; flag_InitEERead input boolean; CD_KEYLESS_ENTRY_FUNCTION parameter uint8; state_CommKeylessEntry output uint8; CD_KEYLESS_ENTRY_DEBOUNCE_MISSING parameter uint16; msgAge_KeylessEntry input uint16; CD_DELUXE_G55_COMM_DEBOUNCE_RUNNING parameter uint16

== stateflow chart MX_Gtwy_Control/PanelInterface/Aux_Ind/Aux1_Indicator ==
  state FLASH1
    state On1
      en:
      ind_Aux1 = ACTIVE;
    state Off1
      en:
      ind_Aux1 = INACTIVE;
    (default) -> On1
    On1 -> Off1 : [after(2,tick)]
    Off1 -> On1 : [after(2,tick)]
  state INACTIVE
    en:
    ind_Aux1 = INACTIVE;
  state ACTIVE
    en:
    ind_Aux1 = ACTIVE;
  state FLASH
    state On
      en:
      ind_Aux1 = ACTIVE;
    state Off
      en:
      ind_Aux1 = INACTIVE;
    (default) -> On
    On -> Off : [after(2,tick)]
    Off -> On : [after(2,tick)]
  (default) -> INACTIVE
  INACTIVE -> FLASH1 [order 2] : [flag_AuxEnableFlash]
  FLASH1 -> INACTIVE : [!flag_AuxEnableFlash]
  INACTIVE -> ACTIVE [order 1] : [state_AuxEnable != INACTIVE]
  ACTIVE -> INACTIVE [order 1] : [state_AuxEnable == INACTIVE]
  ACTIVE -> FLASH [order 2] : [(state_Detent == ERR_DETENT)...
      | && ee_DeluxeG5 != ACTIVE...
      | && CD_DELUXE_GEN5_5_FUNCTION == NON_FUNCTIONAL]
  FLASH -> ACTIVE : [(after(CD_AUX_DETENT_FLASH_TIME,tick)...
      | && state_Detent != ERR_DETENT)...
      | || (state_DetentEnable == ACTIVE)]
  data: state_AuxEnable input uint8; flag_AuxEnableFlash input boolean; state_Detent input uint8; ind_Aux1 output uint8; ACTIVE parameter uint8; INACTIVE parameter uint8; ERR_DETENT parameter uint8; CD_AUX_DETENT_FLASH_TIME parameter uint16; state_DetentEnable input uint8; ee_DeluxeG5 data_store_memory double; CD_DELUXE_GEN5_5_FUNCTION parameter uint8; NON_FUNCTIONAL parameter uint8

== stateflow chart MX_Gtwy_Control/Inputs/Buttons/Delay ==
  state Delay
  state Wait_for_active
    en:
    out = 0;
  note: When we get on the main
      | gauge screen we want
      | a to ignore the autoidle (enter)
      | button for a while or it could change auto
      | idle state if someone is still holding
      | the enter button from a previous screen
  state Active
    en:
    out = 1;
  (default) -> Wait_for_active
  Wait_for_active -> Delay : [in]
  Delay -> Active : [after(50,tick)]
  Active -> Wait_for_active : [!in]
  data: in input boolean; out output boolean

== stateflow chart MX_Gtwy_Control/Inputs/Engine_Oil_Temperature/ErrorDetection ==
  state NON_FUNCTIONAL
    en:state_EOT = INACTIVE;
  state FUNCTIONAL
    state NORMAL
      state DEBOUNCE_NORMAL
        en:debounceCount = 0;
        du:debounceCount++;
      state NORMAL
        en:state_EOT = NORMAL;
      (default) -> DEBOUNCE_NORMAL
      DEBOUNCE_NORMAL -> FUNCTIONAL.NORMAL.NORMAL : [(debounceCount >= CD_EOT_DEBOUNCE_NORMAL)...
          | || (state_EOT == NORMAL)]
    state WAIT_FOR_ECT1
      en:state_EOT = INACTIVE;
    state LOW
      state LOW
        en:state_EOT = LOW;
      state DEBOUNCE
        en:debounceCount = 0;
        du:debounceCount++;
      (default) -> FUNCTIONAL.LOW.DEBOUNCE
      FUNCTIONAL.LOW.DEBOUNCE -> FUNCTIONAL.LOW.LOW : [(debounceCount >= CD_EOT_DEBOUNCE_LOW)...
          | || (state_EOT == LOW)]
    state EXTREMELY_LOW
      state DEBOUNCE
        en:debounceCount = 0;
        du:debounceCount++;
      state EXTREMELY_LOW
        en:state_EOT = EXTREMELY_LOW;
      (default) -> FUNCTIONAL.EXTREMELY_LOW.DEBOUNCE
      FUNCTIONAL.EXTREMELY_LOW.DEBOUNCE -> FUNCTIONAL.EXTREMELY_LOW.EXTREMELY_LOW : [(debounceCount >= CD_EOT_DEBOUNCE_EXTREMELY_LOW)...
          | || (state_EOT == EXTREMELY_LOW)]
    WAIT_FOR_ECT1 -> FUNCTIONAL.NORMAL [order 2] : [(state_ecuECT == NORMAL)...
        | && (ecu_EOT > CD_EOT_LOW)]
    FUNCTIONAL.NORMAL -> FUNCTIONAL.LOW : [(ecu_EOT <= CD_EOT_LOW)]
    FUNCTIONAL.LOW -> FUNCTIONAL.NORMAL [order 1] : [(ecu_EOT > (CD_EOT_LOW + CD_EOT_TEMPERATURE_HYSTERESIS))]
    (default) -> WAIT_FOR_ECT1
    WAIT_FOR_ECT1 -> FUNCTIONAL.LOW [order 1] : [(state_ecuECT == NORMAL)...
        | && (ecu_EOT <= CD_EOT_LOW)]
    FUNCTIONAL.LOW -> FUNCTIONAL.EXTREMELY_LOW [order 2] : [(ecu_EOT <= CD_EOT_EXTREMELY_LOW)]
    FUNCTIONAL.EXTREMELY_LOW -> FUNCTIONAL.LOW : [(ecu_EOT > (CD_EOT_EXTREMELY_LOW + CD_EOT_TEMPERATURE_HYSTERESIS))]
  (default) -> NON_FUNCTIONAL
  NON_FUNCTIONAL -> FUNCTIONAL : [(CD_EOT_FUNCTION != NON_FUNCTIONAL)...
      | && (state_System == SYS_NORMAL)]
  data: ecu_EOT input uint16; state_EOT output uint8; CD_EOT_DEBOUNCE_NORMAL parameter uint16; CD_EOT_DEBOUNCE_LOW parameter uint16; CD_EOT_DEBOUNCE_EXTREMELY_LOW parameter uint16; CD_EOT_LOW parameter uint16; CD_EOT_EXTREMELY_LOW parameter uint16; debounceCount local uint16 =0; CD_EOT_TEMPERATURE_HYSTERESIS parameter uint16; EXTREMELY_LOW parameter uint8; LOW parameter uint8; NON_FUNCTIONAL parameter uint8; NORMAL parameter uint8; state_System input uint8; SYS_NORMAL parameter uint8; state_Engine input uint8; state_ecuECT input uint8; CD_EOT_FUNCTION parameter uint8; INACTIVE parameter uint8

== stateflow chart MX_Gtwy_Control/Outputs/Offset/Offset/Commands ==
  state Inactive
    en:
    icmd_Base = 0;
    icmd_Rod = 0;
  state Active
    state Neutral
      en:
      icmd_Base = 0;
      icmd_Rod = 0;
    state RodActive
      en:
      icmd_Base = 0;
      icmd_Rod = icmd_In;
      du:
      icmd_Rod = icmd_In;
    state BaseActive
      en:
      icmd_Rod = 0;
      icmd_Base = icmd_In;
      du:
      icmd_Base = icmd_In;
    (default) -> Neutral
    Neutral -> RodActive [order 1] : [state_OffsetPot == ROD_ACTIVE]
    BaseActive -> Neutral [order 1] : [state_OffsetPot != BASE_ACTIVE...
        | && state_OffsetPot != ROD_ACTIVE]
    Neutral -> BaseActive [order 2] : [state_OffsetPot == BASE_ACTIVE]
    RodActive -> Neutral [order 1] : [state_OffsetPot != BASE_ACTIVE...
        | && state_OffsetPot != ROD_ACTIVE]
    BaseActive -> RodActive [order 2] : [state_OffsetPot == ROD_ACTIVE]
    RodActive -> BaseActive [order 2] : [state_OffsetPot == BASE_ACTIVE]
  (default) -> Inactive
  Inactive -> Active : [state_Console == ACTIVE...
      | && state_Engine != ENGINE_CRANKING...
      | && (state_Diverter == ACTIVE || state_Diverter == INACTIVE)]
  Active -> Inactive : [state_Console != ACTIVE...
      | || state_Engine == ENGINE_CRANKING...
      | || (state_Diverter != ACTIVE && state_Diverter != INACTIVE)]
  data: icmd_In input fixdt(0,16,12); state_OffsetPot input uint8; state_Engine input uint8; state_Diverter input uint8; ENGINE_CRANKING parameter uint8; icmd_Base output fixdt(0,16,12); BASE_ACTIVE parameter uint8; ROD_ACTIVE parameter uint8; ACTIVE parameter uint8; state_Console input uint8; icmd_Rod output fixdt(0,16,12); INACTIVE parameter uint8

== stateflow chart MX_Gtwy_Control/Outputs/Lights/Lights ==
  settings: executeAtInitialization=1
  state LIGHTS_INACTIVE
    en:
    dout_Lights = INACTIVE;
  state LIGHTS_ACTIVE_RISING1
    en:
    dout_Lights = ACTIVE;
  state LIGHTS_ACTIVE_RISING2
    en:
    dout_Lights = ACTIVE;
  state LIGHTS_ACTIVE
    en:
    state_Buttons_Old = state_Buttons;
    dout_Lights = ACTIVE;
  (default) -> LIGHTS_INACTIVE
  LIGHTS_INACTIVE -> LIGHTS_ACTIVE_RISING1 [order 2] : [((state_VBattery == NORMAL) || (state_VBattery == LOW))...
      | && (CD_LIGHTS_FUNCTION != NON_FUNCTIONAL)...
      | && (button_Lights == ACTIVE)...
      | && (state_LightsOutput == NORMAL)...
      | && (state_LightsRelay == NORMAL)]
  LIGHTS_INACTIVE -> LIGHTS_ACTIVE [order 1] : [((state_VBattery == NORMAL) || (state_VBattery == LOW))...
      | && (CD_LIGHTS_FUNCTION != NON_FUNCTIONAL)...
      | && (state_Buttons == NO_COMMUNICATION)...
      | && (state_LightsOutput == NORMAL)...
      | && (state_LightsRelay == NORMAL)]
  LIGHTS_ACTIVE -> LIGHTS_INACTIVE [order 1] : [(state_Buttons_Old == NO_COMMUNICATION)...
      | && (state_Buttons != NO_COMMUNICATION)...
      | || (!((state_VBattery == NORMAL) || (state_VBattery == LOW)))...
      | || (state_LightsOutput != NORMAL)...
      | || (state_LightsRelay != NORMAL)]
  LIGHTS_ACTIVE_RISING2 -> LIGHTS_INACTIVE : [(button_Lights == INACTIVE)]
  LIGHTS_ACTIVE_RISING1 -> LIGHTS_ACTIVE : [(button_Lights == INACTIVE)]
  LIGHTS_ACTIVE -> LIGHTS_ACTIVE_RISING2 [order 2] : [(button_Lights == ACTIVE)]
  data: state_VBattery input uint8; dout_Lights output boolean; ACTIVE parameter uint8; LOW parameter uint8; NORMAL parameter uint8; INACTIVE parameter uint8; CD_LIGHTS_FUNCTION parameter uint8; state_Buttons input uint8; NO_COMMUNICATION parameter uint8; state_Buttons_Old local uint8; button_Lights input boolean; NON_FUNCTIONAL parameter uint8; state_LightsOutput input uint8; state_LightsRelay input uint8

== stateflow chart MX_Gtwy_Control/Outputs/Starter_old/Starter ==
  state ACTIVE
    en:dout_Starter = ACTIVE
  state INACTIVE
    en:dout_Starter = INACTIVE;
  (default) -> INACTIVE
  INACTIVE -> ACTIVE : [(CD_STARTER_FUNCTION != NON_FUNCTIONAL)...
      | && (state_Engine == ENGINE_CRANKING)...
      | && (state_VBattery != OORL)...
      | && (state_StarterOutput != ERROR_ON)...
      | && (state_StarterRelay != ERROR_ON)...
      | && (error_FuelSec == NORMAL)...
      | && (state_FuelPriOutput == NORMAL)...
      | && (state_FuelPriRelay == NORMAL)]
  ACTIVE -> INACTIVE : [(state_Engine != ENGINE_CRANKING)...
      | || (state_VBattery == OORL)...
      | || (error_FuelSec != NORMAL)...
      | || (state_FuelPriOutput != NORMAL)...
      | || (state_FuelPriRelay != NORMAL)]
  data: state_Engine input uint8; state_VBattery input uint8; dout_Starter output boolean; CD_STARTER_FUNCTION parameter uint8; error_FuelSec input uint8; ACTIVE parameter uint8; ENGINE_CRANKING parameter uint8; INACTIVE parameter uint8; NORMAL parameter uint8; OORL parameter uint8; NON_FUNCTIONAL parameter uint8; state_StarterOutput input uint8; state_StarterRelay input uint8; ERROR_ON parameter uint8; state_FuelPriOutput input uint8; state_FuelPriRelay input uint8

== stateflow chart MX_Gtwy_Control/Outputs/FuelPri_HVAC/HVAC_Relay/RelaySolenoid ==
  state INACTIVE
    en:dout_HVACRelay = INACTIVE;
  state STARTUP_ERROR_ON_CHECK
    en:dout_HVACRelay = INACTIVE;
    en:debounceCount = 0;
    du:debounceCount++;
  state ACTIVE
    en:dout_HVACRelay = ACTIVE;
  (default) -> STARTUP_ERROR_ON_CHECK
  STARTUP_ERROR_ON_CHECK -> INACTIVE [order 1] : [(debounceCount >= CD_HVAC_RELAY_DEBOUNCE_ERROR_ON + 100)]
  STARTUP_ERROR_ON_CHECK -> ACTIVE [order 2] : [(CD_HVAC_RELAY_FUNCTION != NON_FUNCTIONAL)...
      | && (state_Engine == ENGINE_RUNNING)...
      | && (state_OutputError == NORMAL)...
      | && (state_System == SYS_NORMAL)]
  ACTIVE -> INACTIVE : [(state_Engine == ENGINE_CRANKING)...
      | || (state_OutputError != NORMAL)...
      | || (state_System != SYS_NORMAL)]
  INACTIVE -> ACTIVE : [(CD_HVAC_RELAY_FUNCTION != NON_FUNCTIONAL)...
      | && ((state_Engine != ENGINE_CRANKING) || (state_Engine == ENGINE_RUNNING))...
      | && (state_OutputError == NORMAL)...
      | && (state_System == SYS_NORMAL)]
  data: dout_HVACRelay output boolean; state_OutputError input uint8; state_Engine input uint8; ACTIVE parameter uint8; ENGINE_CRANKING parameter uint8; INACTIVE parameter uint8; NORMAL parameter uint8; NON_FUNCTIONAL parameter uint8; CD_HVAC_RELAY_FUNCTION parameter uint8; state_System input uint8; SYS_NORMAL parameter uint8; debounceCount local uint16; CD_HVAC_RELAY_DEBOUNCE_ERROR_ON parameter uint16; ENGINE_RUNNING parameter uint8
